simulink model "BaseEngineController_A02"  (Simulink 7.7)
settings: Solver=FixedStepDiscrete  StartTime=0.0  StopTime=10.0  RelTol=1e-3
PreLoadFcn:
  | try, eval([bdroot '_setup']); catch, eval('model_setup', ''); end
InitFcn:
  | try, eval([bdroot '_setup']); catch, eval('model_setup', ''); end
undefined symbols (not defined in the file or in its folder): ECU555, High, motohawk_build_version, motohawk_get_faults, motohawk_trunc_name, motohawk_vardec_config_group, motohawk_vardec_path, True
libraries: MotoHawk_lib x1145, AutoDoc_lib x786, motohawk_control_primitives_lib x114, motohawk_engine_lib x19, motohawk_annotations_lib x12, simulink x11, simulink_extras x1
(part 13 of 23)

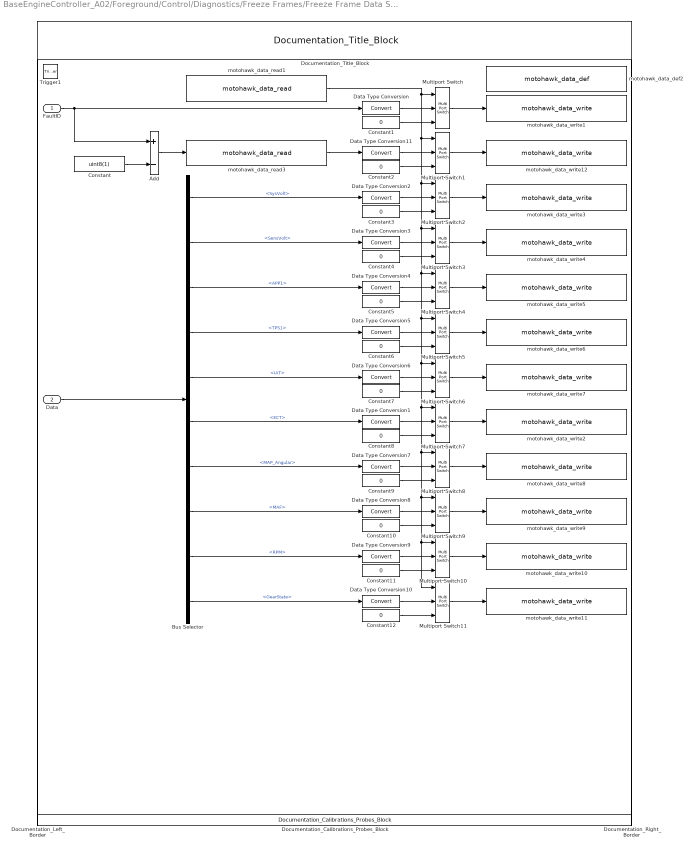
== system BaseEngineController_A02/Foreground/Control/Diagnostics/Freeze Frames/Freeze Frame Data Storage1 ==
  FaultID : Inport
  Data : Inport  Port=2
  Trigger1 : TriggerPort
  Add : Sum  Inputs=+-  OutDataTypeStr=Inherit: Same as first input
  Bus Selector : BusSelector  OutputSignals=SynthSensors.Raw.LinearSensors.SysVolt,SynthSensors.Raw.LinearSensors.SensVolt,SynthSensors.Raw.PotSensors.APP1,SynthSensors.Raw.PotSensors.TPS1,SynthSensors.Raw.NonLinearSensors.IAT,SynthSensors.Raw.NonLinearSensors.ECT,SynthSensors.Raw.LinearSensors.MAP_Angular,SynthSensors.Raw.Digital.MAF,SynthSensors.Raw.RPM,SynthSensors.Raw.Digital.GearState
  Constant : Constant  Value=uint8(1)
  Constant{1..12} (12 alike) : Constant  Value=0  OutDataTypeStr=Inherit: Inherit via back propagation
  Data Type Conversion{,1..11} (12 alike) : DataTypeConversion
  Documentation_Calibrations_Probes_Block : AutoDoc_lib/Documentation_Calibrations_Probes_Block
  Documentation_Left_Border : AutoDoc_lib/Documentation_Left_Border  layout=Portrait
  Documentation_Right_Border : AutoDoc_lib/Documentation_Right_Border  layout=Portrait
  Documentation_Title_Block : AutoDoc_lib/Documentation_Title_Block
  Multiport Switch{,1..11} (12 alike) : MultiPortSwitch  DataPortOrder=Zero-based contiguous  Inputs=2  zeroidx=on  InputSameDT=on
  motohawk_data_def2 : MotoHawk_lib/Data Storage Blocks/motohawk_data_def  nam=['FF' num2str(FFnum)]  container_nam='FreezeFramesContainer'  data=struct('Name', int32(0), 'Code', int32(0), 'Var1', int32(0), 'Var2', int32(0), 'Var3', int32(0), 'Var4', int32(0), 'Var5', int32(0), 'Var6', int32(0), 'Var7', int32(0), 'Var8', int32(0), 'Var9', int32(0), 'Var10', int32(0))  typ=struct  struct_nam='FreezeFrames'  storage=NonVolatile  outputRef=off  use_vardec=off  window=Display  read_level=1  write_level=(Read Only)  ovr_cals=on  view_as=Number  enum_struct={'Disabled', 'Enabled'}  vector_style=Wide Row  use_help_units=off  help=''  units=''  use_header_enum=off  row_header_enum={}  col_header_enum={}  use_min_max=off  min_val=-inf  max_val=inf  use_precision=off  precision=''  gain=1  offset=0  exp=1  use_mototune_group=off  mototune_group=''  eval_name=FF1  data_stores=motohawk_sfun_data_def_data_stores  struct_instances=motohawk_sfun_data_def_struct_instances  vardecs=motohawk_sfun_data_def_vardecs
  motohawk_data_read1 : MotoHawk_lib/Data Storage Blocks/motohawk_data_read  source=Lookup By Name  structure=Scalar  operation=Read scalar from location by index  sz_vec=3  sz_row=2  sz_col=3  typ=boolean  nam='ClearFreezeFrames'  checkNull=on  struct_inst_name='Struct1'  struct_nam='MyStruct'
  motohawk_data_read3 : MotoHawk_lib/Data Storage Blocks/motohawk_data_read  source=Lookup By Name  structure=Vector  operation=Read scalar from location by index  sz_vec=3  sz_row=2  sz_col=3  typ=uint16  nam='FltCodes'  checkNull=on  struct_inst_name='Struct1'  struct_nam='MyStruct'
  motohawk_data_write1 : MotoHawk_lib/Data Storage Blocks/motohawk_data_write  source=Lookup By Name in Structure  structure=Scalar  operation=Write entire data structure at once  sz_vec=len  sz_row=10  sz_col=1  typ=int32  nam='Name'  checkNull=on  write_read_only=off  struct_inst_name=['FF' num2str(FFnum)]  struct_name='FreezeFrames'
  motohawk_data_write10 : MotoHawk_lib/Data Storage Blocks/motohawk_data_write  source=Lookup By Name in Structure  structure=Scalar  operation=Write entire data structure at once  sz_vec=len  sz_row=10  sz_col=1  typ=int32  nam='Var9'  checkNull=on  write_read_only=off  struct_inst_name=['FF' num2str(FFnum)]  struct_name='FreezeFrames'
  motohawk_data_write11 : MotoHawk_lib/Data Storage Blocks/motohawk_data_write  source=Lookup By Name in Structure  structure=Scalar  operation=Write entire data structure at once  sz_vec=len  sz_row=10  sz_col=1  typ=int32  nam='Var10'  checkNull=on  write_read_only=off  struct_inst_name=['FF' num2str(FFnum)]  struct_name='FreezeFrames'
  motohawk_data_write12 : MotoHawk_lib/Data Storage Blocks/motohawk_data_write  source=Lookup By Name in Structure  structure=Scalar  operation=Write entire data structure at once  sz_vec=len  sz_row=10  sz_col=1  typ=int32  nam='Code'  checkNull=on  write_read_only=off  struct_inst_name=['FF' num2str(FFnum)]  struct_name='FreezeFrames'
  motohawk_data_write2 : MotoHawk_lib/Data Storage Blocks/motohawk_data_write  source=Lookup By Name in Structure  structure=Scalar  operation=Write entire data structure at once  sz_vec=len  sz_row=10  sz_col=1  typ=int32  nam='Var6'  checkNull=on  write_read_only=off  struct_inst_name=['FF' num2str(FFnum)]  struct_name='FreezeFrames'
  motohawk_data_write3 : MotoHawk_lib/Data Storage Blocks/motohawk_data_write  source=Lookup By Name in Structure  structure=Scalar  operation=Write entire data structure at once  sz_vec=len  sz_row=10  sz_col=1  typ=int32  nam='Var1'  checkNull=on  write_read_only=off  struct_inst_name=['FF' num2str(FFnum)]  struct_name='FreezeFrames'
  motohawk_data_write4 : MotoHawk_lib/Data Storage Blocks/motohawk_data_write  source=Lookup By Name in Structure  structure=Scalar  operation=Write entire data structure at once  sz_vec=len  sz_row=10  sz_col=1  typ=int32  nam='Var2'  checkNull=on  write_read_only=off  struct_inst_name=['FF' num2str(FFnum)]  struct_name='FreezeFrames'
  motohawk_data_write5 : MotoHawk_lib/Data Storage Blocks/motohawk_data_write  source=Lookup By Name in Structure  structure=Scalar  operation=Write entire data structure at once  sz_vec=len  sz_row=10  sz_col=1  typ=int32  nam='Var3'  checkNull=on  write_read_only=off  struct_inst_name=['FF' num2str(FFnum)]  struct_name='FreezeFrames'
  motohawk_data_write6 : MotoHawk_lib/Data Storage Blocks/motohawk_data_write  source=Lookup By Name in Structure  structure=Scalar  operation=Write entire data structure at once  sz_vec=len  sz_row=10  sz_col=1  typ=int32  nam='Var4'  checkNull=on  write_read_only=off  struct_inst_name=['FF' num2str(FFnum)]  struct_name='FreezeFrames'
  motohawk_data_write7 : MotoHawk_lib/Data Storage Blocks/motohawk_data_write  source=Lookup By Name in Structure  structure=Scalar  operation=Write entire data structure at once  sz_vec=len  sz_row=10  sz_col=1  typ=int32  nam='Var5'  checkNull=on  write_read_only=off  struct_inst_name=['FF' num2str(FFnum)]  struct_name='FreezeFrames'
  motohawk_data_write8 : MotoHawk_lib/Data Storage Blocks/motohawk_data_write  source=Lookup By Name in Structure  structure=Scalar  operation=Write entire data structure at once  sz_vec=len  sz_row=10  sz_col=1  typ=int32  nam='Var7'  checkNull=on  write_read_only=off  struct_inst_name=['FF' num2str(FFnum)]  struct_name='FreezeFrames'
  motohawk_data_write9 : MotoHawk_lib/Data Storage Blocks/motohawk_data_write  source=Lookup By Name in Structure  structure=Scalar  operation=Write entire data structure at once  sz_vec=len  sz_row=10  sz_col=1  typ=int32  nam='Var8'  checkNull=on  write_read_only=off  struct_inst_name=['FF' num2str(FFnum)]  struct_name='FreezeFrames'
  wires:
  Data Type Conversion10/1 -> Multiport Switch11/2
  Data Type Conversion9/1 -> Multiport Switch10/2
  Data Type Conversion8/1 -> Multiport Switch9/2
  Data Type Conversion7/1 -> Multiport Switch8/2
  Data Type Conversion1/1 -> Multiport Switch7/2
  Data Type Conversion6/1 -> Multiport Switch6/2
  Data Type Conversion5/1 -> Multiport Switch5/2
  Data Type Conversion4/1 -> Multiport Switch4/2
  Data Type Conversion3/1 -> Multiport Switch3/2
  Data Type Conversion2/1 -> Multiport Switch2/2
  Multiport Switch11/1 -> motohawk_data_write11/1
  Multiport Switch10/1 -> motohawk_data_write10/1
  Multiport Switch9/1 -> motohawk_data_write9/1
  Multiport Switch8/1 -> motohawk_data_write8/1
  Multiport Switch7/1 -> motohawk_data_write2/1
  Multiport Switch6/1 -> motohawk_data_write7/1
  Multiport Switch5/1 -> motohawk_data_write6/1
  Multiport Switch4/1 -> motohawk_data_write5/1
  Multiport Switch3/1 -> motohawk_data_write4/1
  Multiport Switch2/1 -> motohawk_data_write3/1
  Constant12/1 -> Multiport Switch11/3
  Constant11/1 -> Multiport Switch10/3
  Constant10/1 -> Multiport Switch9/3
  Constant9/1 -> Multiport Switch8/3
  Constant8/1 -> Multiport Switch7/3
  Constant7/1 -> Multiport Switch6/3
  Constant6/1 -> Multiport Switch5/3
  Constant5/1 -> Multiport Switch4/3
  Constant4/1 -> Multiport Switch3/3
  Constant3/1 -> Multiport Switch2/3
  Data Type Conversion11/1 -> Multiport Switch1/2
  Constant2/1 -> Multiport Switch1/3
  Multiport Switch1/1 -> motohawk_data_write12/1
  Multiport Switch/1 -> motohawk_data_write1/1
  motohawk_data_read1/1 -> Multiport Switch10/1, Multiport Switch11/1, Multiport Switch9/1, Multiport Switch8/1, Multiport Switch7/1, Multiport Switch6/1, Multiport Switch5/1, Multiport Switch4/1, Multiport Switch3/1, Multiport Switch2/1, Multiport Switch1/1, Multiport Switch/1
  Constant1/1 -> Multiport Switch/3
  Data Type Conversion/1 -> Multiport Switch/2
  Add/1 -> motohawk_data_read3/1
  Constant/1 -> Add/2
  motohawk_data_read3/1 -> Data Type Conversion11/1
  Bus Selector/10 -> Data Type Conversion10/1  "<GearState>"
  Bus Selector/9 -> Data Type Conversion9/1  "<RPM>"
  Bus Selector/8 -> Data Type Conversion8/1  "<MAF>"
  Bus Selector/7 -> Data Type Conversion7/1  "<MAP_Angular>"
  Bus Selector/6 -> Data Type Conversion1/1  "<ECT>"
  Data/1 -> Bus Selector/1
  Bus Selector/5 -> Data Type Conversion6/1  "<IAT>"
  Bus Selector/4 -> Data Type Conversion5/1  "<TPS1>"
  Bus Selector/3 -> Data Type Conversion4/1  "<APP1>"
  Bus Selector/2 -> Data Type Conversion3/1  "<SensVolt>"
  Bus Selector/1 -> Data Type Conversion2/1  "<SysVolt>"
  FaultID/1 -> Add/1, Data Type Conversion/1

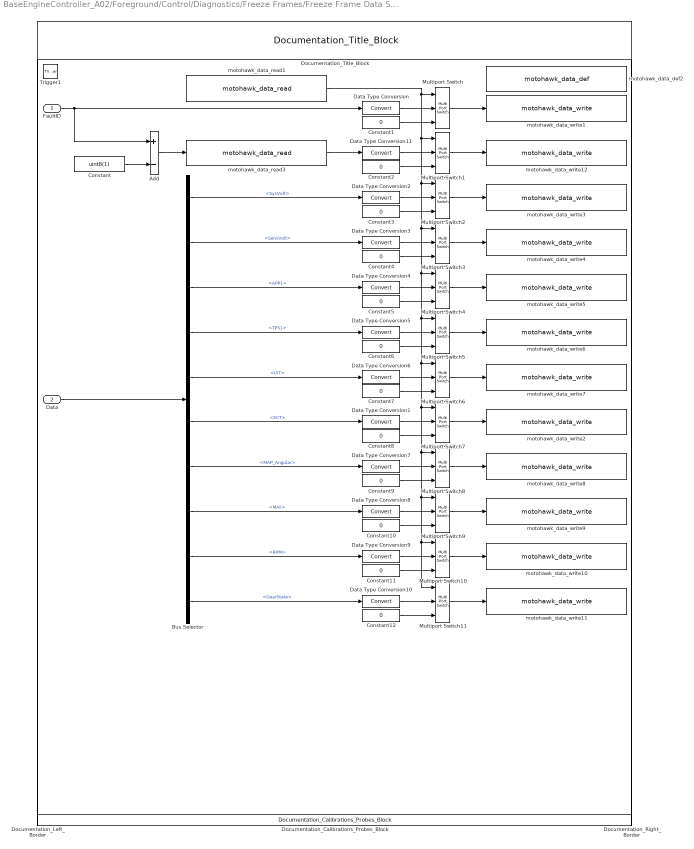
== system BaseEngineController_A02/Foreground/Control/Diagnostics/Freeze Frames/Freeze Frame Data Storage2 ==
  FaultID : Inport
  Data : Inport  Port=2
  Trigger1 : TriggerPort
  Add : Sum  Inputs=+-  OutDataTypeStr=Inherit: Same as first input
  Bus Selector : BusSelector  OutputSignals=SynthSensors.Raw.LinearSensors.SysVolt,SynthSensors.Raw.LinearSensors.SensVolt,SynthSensors.Raw.PotSensors.APP1,SynthSensors.Raw.PotSensors.TPS1,SynthSensors.Raw.NonLinearSensors.IAT,SynthSensors.Raw.NonLinearSensors.ECT,SynthSensors.Raw.LinearSensors.MAP_Angular,SynthSensors.Raw.Digital.MAF,SynthSensors.Raw.RPM,SynthSensors.Raw.Digital.GearState
  Constant : Constant  Value=uint8(1)
  Constant{1..12} (12 alike) : Constant  Value=0  OutDataTypeStr=Inherit: Inherit via back propagation
  Data Type Conversion{,1..11} (12 alike) : DataTypeConversion
  Documentation_Calibrations_Probes_Block : AutoDoc_lib/Documentation_Calibrations_Probes_Block
  Documentation_Left_Border : AutoDoc_lib/Documentation_Left_Border  layout=Portrait
  Documentation_Right_Border : AutoDoc_lib/Documentation_Right_Border  layout=Portrait
  Documentation_Title_Block : AutoDoc_lib/Documentation_Title_Block
  Multiport Switch{,1..11} (12 alike) : MultiPortSwitch  DataPortOrder=Zero-based contiguous  Inputs=2  zeroidx=on  InputSameDT=on
  motohawk_data_def2 : MotoHawk_lib/Data Storage Blocks/motohawk_data_def  nam=['FF' num2str(FFnum)]  container_nam='FreezeFramesContainer'  data=struct('Name', int32(0), 'Code', int32(0), 'Var1', int32(0), 'Var2', int32(0), 'Var3', int32(0), 'Var4', int32(0), 'Var5', int32(0), 'Var6', int32(0), 'Var7', int32(0), 'Var8', int32(0), 'Var9', int32(0), 'Var10', int32(0))  typ=struct  struct_nam='FreezeFrames'  storage=NonVolatile  outputRef=off  use_vardec=off  window=Display  read_level=1  write_level=(Read Only)  ovr_cals=on  view_as=Number  enum_struct={'Disabled', 'Enabled'}  vector_style=Wide Row  use_help_units=off  help=''  units=''  use_header_enum=off  row_header_enum={}  col_header_enum={}  use_min_max=off  min_val=-inf  max_val=inf  use_precision=off  precision=''  gain=1  offset=0  exp=1  use_mototune_group=off  mototune_group=''  eval_name=FF1  data_stores=motohawk_sfun_data_def_data_stores  struct_instances=motohawk_sfun_data_def_struct_instances  vardecs=motohawk_sfun_data_def_vardecs
  motohawk_data_read1 : MotoHawk_lib/Data Storage Blocks/motohawk_data_read  source=Lookup By Name  structure=Scalar  operation=Read scalar from location by index  sz_vec=3  sz_row=2  sz_col=3  typ=boolean  nam='ClearFreezeFrames'  checkNull=on  struct_inst_name='Struct1'  struct_nam='MyStruct'
  motohawk_data_read3 : MotoHawk_lib/Data Storage Blocks/motohawk_data_read  source=Lookup By Name  structure=Vector  operation=Read scalar from location by index  sz_vec=3  sz_row=2  sz_col=3  typ=uint16  nam='FltCodes'  checkNull=on  struct_inst_name='Struct1'  struct_nam='MyStruct'
  motohawk_data_write1 : MotoHawk_lib/Data Storage Blocks/motohawk_data_write  source=Lookup By Name in Structure  structure=Scalar  operation=Write entire data structure at once  sz_vec=len  sz_row=10  sz_col=1  typ=int32  nam='Name'  checkNull=on  write_read_only=off  struct_inst_name=['FF' num2str(FFnum)]  struct_name='FreezeFrames'
  motohawk_data_write10 : MotoHawk_lib/Data Storage Blocks/motohawk_data_write  source=Lookup By Name in Structure  structure=Scalar  operation=Write entire data structure at once  sz_vec=len  sz_row=10  sz_col=1  typ=int32  nam='Var9'  checkNull=on  write_read_only=off  struct_inst_name=['FF' num2str(FFnum)]  struct_name='FreezeFrames'
  motohawk_data_write11 : MotoHawk_lib/Data Storage Blocks/motohawk_data_write  source=Lookup By Name in Structure  structure=Scalar  operation=Write entire data structure at once  sz_vec=len  sz_row=10  sz_col=1  typ=int32  nam='Var10'  checkNull=on  write_read_only=off  struct_inst_name=['FF' num2str(FFnum)]  struct_name='FreezeFrames'
  motohawk_data_write12 : MotoHawk_lib/Data Storage Blocks/motohawk_data_write  source=Lookup By Name in Structure  structure=Scalar  operation=Write entire data structure at once  sz_vec=len  sz_row=10  sz_col=1  typ=int32  nam='Code'  checkNull=on  write_read_only=off  struct_inst_name=['FF' num2str(FFnum)]  struct_name='FreezeFrames'
  motohawk_data_write2 : MotoHawk_lib/Data Storage Blocks/motohawk_data_write  source=Lookup By Name in Structure  structure=Scalar  operation=Write entire data structure at once  sz_vec=len  sz_row=10  sz_col=1  typ=int32  nam='Var6'  checkNull=on  write_read_only=off  struct_inst_name=['FF' num2str(FFnum)]  struct_name='FreezeFrames'
  motohawk_data_write3 : MotoHawk_lib/Data Storage Blocks/motohawk_data_write  source=Lookup By Name in Structure  structure=Scalar  operation=Write entire data structure at once  sz_vec=len  sz_row=10  sz_col=1  typ=int32  nam='Var1'  checkNull=on  write_read_only=off  struct_inst_name=['FF' num2str(FFnum)]  struct_name='FreezeFrames'
  motohawk_data_write4 : MotoHawk_lib/Data Storage Blocks/motohawk_data_write  source=Lookup By Name in Structure  structure=Scalar  operation=Write entire data structure at once  sz_vec=len  sz_row=10  sz_col=1  typ=int32  nam='Var2'  checkNull=on  write_read_only=off  struct_inst_name=['FF' num2str(FFnum)]  struct_name='FreezeFrames'
  motohawk_data_write5 : MotoHawk_lib/Data Storage Blocks/motohawk_data_write  source=Lookup By Name in Structure  structure=Scalar  operation=Write entire data structure at once  sz_vec=len  sz_row=10  sz_col=1  typ=int32  nam='Var3'  checkNull=on  write_read_only=off  struct_inst_name=['FF' num2str(FFnum)]  struct_name='FreezeFrames'
  motohawk_data_write6 : MotoHawk_lib/Data Storage Blocks/motohawk_data_write  source=Lookup By Name in Structure  structure=Scalar  operation=Write entire data structure at once  sz_vec=len  sz_row=10  sz_col=1  typ=int32  nam='Var4'  checkNull=on  write_read_only=off  struct_inst_name=['FF' num2str(FFnum)]  struct_name='FreezeFrames'
  motohawk_data_write7 : MotoHawk_lib/Data Storage Blocks/motohawk_data_write  source=Lookup By Name in Structure  structure=Scalar  operation=Write entire data structure at once  sz_vec=len  sz_row=10  sz_col=1  typ=int32  nam='Var5'  checkNull=on  write_read_only=off  struct_inst_name=['FF' num2str(FFnum)]  struct_name='FreezeFrames'
  motohawk_data_write8 : MotoHawk_lib/Data Storage Blocks/motohawk_data_write  source=Lookup By Name in Structure  structure=Scalar  operation=Write entire data structure at once  sz_vec=len  sz_row=10  sz_col=1  typ=int32  nam='Var7'  checkNull=on  write_read_only=off  struct_inst_name=['FF' num2str(FFnum)]  struct_name='FreezeFrames'
  motohawk_data_write9 : MotoHawk_lib/Data Storage Blocks/motohawk_data_write  source=Lookup By Name in Structure  structure=Scalar  operation=Write entire data structure at once  sz_vec=len  sz_row=10  sz_col=1  typ=int32  nam='Var8'  checkNull=on  write_read_only=off  struct_inst_name=['FF' num2str(FFnum)]  struct_name='FreezeFrames'
  wires:
  FaultID/1 -> Data Type Conversion/1, Add/1
  Bus Selector/1 -> Data Type Conversion2/1  "<SysVolt>"
  Bus Selector/2 -> Data Type Conversion3/1  "<SensVolt>"
  Bus Selector/3 -> Data Type Conversion4/1  "<APP1>"
  Bus Selector/4 -> Data Type Conversion5/1  "<TPS1>"
  Bus Selector/5 -> Data Type Conversion6/1  "<IAT>"
  Data/1 -> Bus Selector/1
  Bus Selector/6 -> Data Type Conversion1/1  "<ECT>"
  Bus Selector/7 -> Data Type Conversion7/1  "<MAP_Angular>"
  Bus Selector/8 -> Data Type Conversion8/1  "<MAF>"
  Bus Selector/9 -> Data Type Conversion9/1  "<RPM>"
  Bus Selector/10 -> Data Type Conversion10/1  "<GearState>"
  motohawk_data_read3/1 -> Data Type Conversion11/1
  Constant/1 -> Add/2
  Add/1 -> motohawk_data_read3/1
  Data Type Conversion/1 -> Multiport Switch/2
  Constant1/1 -> Multiport Switch/3
  motohawk_data_read1/1 -> Multiport Switch/1, Multiport Switch1/1, Multiport Switch2/1, Multiport Switch3/1, Multiport Switch4/1, Multiport Switch5/1, Multiport Switch6/1, Multiport Switch7/1, Multiport Switch8/1, Multiport Switch9/1, Multiport Switch11/1, Multiport Switch10/1
  Multiport Switch/1 -> motohawk_data_write1/1
  Multiport Switch1/1 -> motohawk_data_write12/1
  Constant2/1 -> Multiport Switch1/3
  Data Type Conversion11/1 -> Multiport Switch1/2
  Constant3/1 -> Multiport Switch2/3
  Constant4/1 -> Multiport Switch3/3
  Constant5/1 -> Multiport Switch4/3
  Constant6/1 -> Multiport Switch5/3
  Constant7/1 -> Multiport Switch6/3
  Constant8/1 -> Multiport Switch7/3
  Constant9/1 -> Multiport Switch8/3
  Constant10/1 -> Multiport Switch9/3
  Constant11/1 -> Multiport Switch10/3
  Constant12/1 -> Multiport Switch11/3
  Multiport Switch2/1 -> motohawk_data_write3/1
  Multiport Switch3/1 -> motohawk_data_write4/1
  Multiport Switch4/1 -> motohawk_data_write5/1
  Multiport Switch5/1 -> motohawk_data_write6/1
  Multiport Switch6/1 -> motohawk_data_write7/1
  Multiport Switch7/1 -> motohawk_data_write2/1
  Multiport Switch8/1 -> motohawk_data_write8/1
  Multiport Switch9/1 -> motohawk_data_write9/1
  Multiport Switch10/1 -> motohawk_data_write10/1
  Multiport Switch11/1 -> motohawk_data_write11/1
  Data Type Conversion2/1 -> Multiport Switch2/2
  Data Type Conversion3/1 -> Multiport Switch3/2
  Data Type Conversion4/1 -> Multiport Switch4/2
  Data Type Conversion5/1 -> Multiport Switch5/2
  Data Type Conversion6/1 -> Multiport Switch6/2
  Data Type Conversion1/1 -> Multiport Switch7/2
  Data Type Conversion7/1 -> Multiport Switch8/2
  Data Type Conversion8/1 -> Multiport Switch9/2
  Data Type Conversion9/1 -> Multiport Switch10/2
  Data Type Conversion10/1 -> Multiport Switch11/2

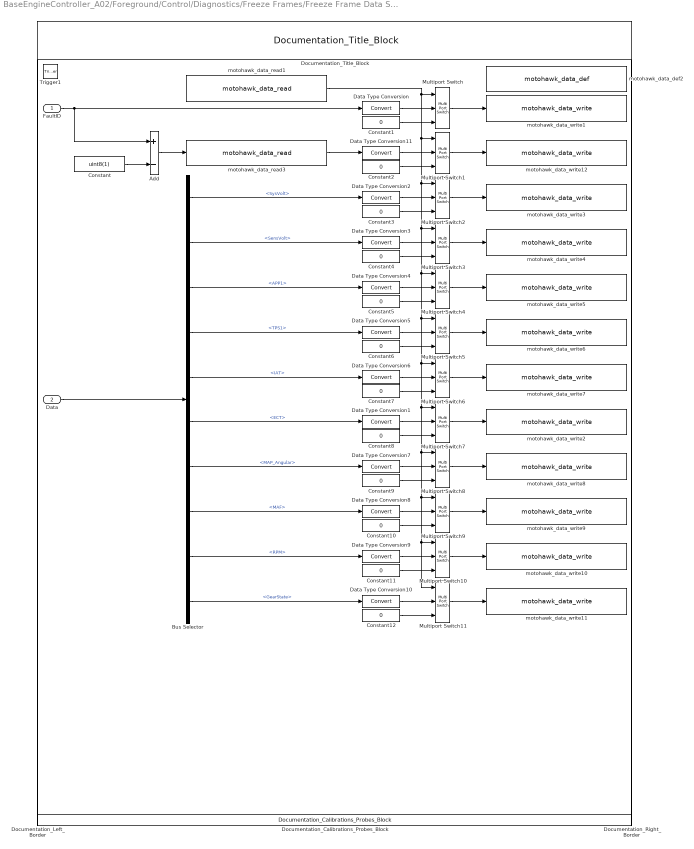
== system BaseEngineController_A02/Foreground/Control/Diagnostics/Freeze Frames/Freeze Frame Data Storage3 ==
  FaultID : Inport
  Data : Inport  Port=2
  Trigger1 : TriggerPort
  Add : Sum  Inputs=+-  OutDataTypeStr=Inherit: Same as first input
  Bus Selector : BusSelector  OutputSignals=SynthSensors.Raw.LinearSensors.SysVolt,SynthSensors.Raw.LinearSensors.SensVolt,SynthSensors.Raw.PotSensors.APP1,SynthSensors.Raw.PotSensors.TPS1,SynthSensors.Raw.NonLinearSensors.IAT,SynthSensors.Raw.NonLinearSensors.ECT,SynthSensors.Raw.LinearSensors.MAP_Angular,SynthSensors.Raw.Digital.MAF,SynthSensors.Raw.RPM,SynthSensors.Raw.Digital.GearState
  Constant : Constant  Value=uint8(1)
  Constant{1..12} (12 alike) : Constant  Value=0  OutDataTypeStr=Inherit: Inherit via back propagation
  Data Type Conversion{,1..11} (12 alike) : DataTypeConversion
  Documentation_Calibrations_Probes_Block : AutoDoc_lib/Documentation_Calibrations_Probes_Block
  Documentation_Left_Border : AutoDoc_lib/Documentation_Left_Border  layout=Portrait
  Documentation_Right_Border : AutoDoc_lib/Documentation_Right_Border  layout=Portrait
  Documentation_Title_Block : AutoDoc_lib/Documentation_Title_Block
  Multiport Switch{,1..11} (12 alike) : MultiPortSwitch  DataPortOrder=Zero-based contiguous  Inputs=2  zeroidx=on  InputSameDT=on
  motohawk_data_def2 : MotoHawk_lib/Data Storage Blocks/motohawk_data_def  nam=['FF' num2str(FFnum)]  container_nam='FreezeFramesContainer'  data=struct('Name', int32(0), 'Code', int32(0), 'Var1', int32(0), 'Var2', int32(0), 'Var3', int32(0), 'Var4', int32(0), 'Var5', int32(0), 'Var6', int32(0), 'Var7', int32(0), 'Var8', int32(0), 'Var9', int32(0), 'Var10', int32(0))  typ=struct  struct_nam='FreezeFrames'  storage=NonVolatile  outputRef=off  use_vardec=off  window=Display  read_level=1  write_level=(Read Only)  ovr_cals=on  view_as=Number  enum_struct={'Disabled', 'Enabled'}  vector_style=Wide Row  use_help_units=off  help=''  units=''  use_header_enum=off  row_header_enum={}  col_header_enum={}  use_min_max=off  min_val=-inf  max_val=inf  use_precision=off  precision=''  gain=1  offset=0  exp=1  use_mototune_group=off  mototune_group=''  eval_name=FF1  data_stores=motohawk_sfun_data_def_data_stores  struct_instances=motohawk_sfun_data_def_struct_instances  vardecs=motohawk_sfun_data_def_vardecs
  motohawk_data_read1 : MotoHawk_lib/Data Storage Blocks/motohawk_data_read  source=Lookup By Name  structure=Scalar  operation=Read scalar from location by index  sz_vec=3  sz_row=2  sz_col=3  typ=boolean  nam='ClearFreezeFrames'  checkNull=on  struct_inst_name='Struct1'  struct_nam='MyStruct'
  motohawk_data_read3 : MotoHawk_lib/Data Storage Blocks/motohawk_data_read  source=Lookup By Name  structure=Vector  operation=Read scalar from location by index  sz_vec=3  sz_row=2  sz_col=3  typ=uint16  nam='FltCodes'  checkNull=on  struct_inst_name='Struct1'  struct_nam='MyStruct'
  motohawk_data_write1 : MotoHawk_lib/Data Storage Blocks/motohawk_data_write  source=Lookup By Name in Structure  structure=Scalar  operation=Write entire data structure at once  sz_vec=len  sz_row=10  sz_col=1  typ=int32  nam='Name'  checkNull=on  write_read_only=off  struct_inst_name=['FF' num2str(FFnum)]  struct_name='FreezeFrames'
  motohawk_data_write10 : MotoHawk_lib/Data Storage Blocks/motohawk_data_write  source=Lookup By Name in Structure  structure=Scalar  operation=Write entire data structure at once  sz_vec=len  sz_row=10  sz_col=1  typ=int32  nam='Var9'  checkNull=on  write_read_only=off  struct_inst_name=['FF' num2str(FFnum)]  struct_name='FreezeFrames'
  motohawk_data_write11 : MotoHawk_lib/Data Storage Blocks/motohawk_data_write  source=Lookup By Name in Structure  structure=Scalar  operation=Write entire data structure at once  sz_vec=len  sz_row=10  sz_col=1  typ=int32  nam='Var10'  checkNull=on  write_read_only=off  struct_inst_name=['FF' num2str(FFnum)]  struct_name='FreezeFrames'
  motohawk_data_write12 : MotoHawk_lib/Data Storage Blocks/motohawk_data_write  source=Lookup By Name in Structure  structure=Scalar  operation=Write entire data structure at once  sz_vec=len  sz_row=10  sz_col=1  typ=int32  nam='Code'  checkNull=on  write_read_only=off  struct_inst_name=['FF' num2str(FFnum)]  struct_name='FreezeFrames'
  motohawk_data_write2 : MotoHawk_lib/Data Storage Blocks/motohawk_data_write  source=Lookup By Name in Structure  structure=Scalar  operation=Write entire data structure at once  sz_vec=len  sz_row=10  sz_col=1  typ=int32  nam='Var6'  checkNull=on  write_read_only=off  struct_inst_name=['FF' num2str(FFnum)]  struct_name='FreezeFrames'
  motohawk_data_write3 : MotoHawk_lib/Data Storage Blocks/motohawk_data_write  source=Lookup By Name in Structure  structure=Scalar  operation=Write entire data structure at once  sz_vec=len  sz_row=10  sz_col=1  typ=int32  nam='Var1'  checkNull=on  write_read_only=off  struct_inst_name=['FF' num2str(FFnum)]  struct_name='FreezeFrames'
  motohawk_data_write4 : MotoHawk_lib/Data Storage Blocks/motohawk_data_write  source=Lookup By Name in Structure  structure=Scalar  operation=Write entire data structure at once  sz_vec=len  sz_row=10  sz_col=1  typ=int32  nam='Var2'  checkNull=on  write_read_only=off  struct_inst_name=['FF' num2str(FFnum)]  struct_name='FreezeFrames'
  motohawk_data_write5 : MotoHawk_lib/Data Storage Blocks/motohawk_data_write  source=Lookup By Name in Structure  structure=Scalar  operation=Write entire data structure at once  sz_vec=len  sz_row=10  sz_col=1  typ=int32  nam='Var3'  checkNull=on  write_read_only=off  struct_inst_name=['FF' num2str(FFnum)]  struct_name='FreezeFrames'
  motohawk_data_write6 : MotoHawk_lib/Data Storage Blocks/motohawk_data_write  source=Lookup By Name in Structure  structure=Scalar  operation=Write entire data structure at once  sz_vec=len  sz_row=10  sz_col=1  typ=int32  nam='Var4'  checkNull=on  write_read_only=off  struct_inst_name=['FF' num2str(FFnum)]  struct_name='FreezeFrames'
  motohawk_data_write7 : MotoHawk_lib/Data Storage Blocks/motohawk_data_write  source=Lookup By Name in Structure  structure=Scalar  operation=Write entire data structure at once  sz_vec=len  sz_row=10  sz_col=1  typ=int32  nam='Var5'  checkNull=on  write_read_only=off  struct_inst_name=['FF' num2str(FFnum)]  struct_name='FreezeFrames'
  motohawk_data_write8 : MotoHawk_lib/Data Storage Blocks/motohawk_data_write  source=Lookup By Name in Structure  structure=Scalar  operation=Write entire data structure at once  sz_vec=len  sz_row=10  sz_col=1  typ=int32  nam='Var7'  checkNull=on  write_read_only=off  struct_inst_name=['FF' num2str(FFnum)]  struct_name='FreezeFrames'
  motohawk_data_write9 : MotoHawk_lib/Data Storage Blocks/motohawk_data_write  source=Lookup By Name in Structure  structure=Scalar  operation=Write entire data structure at once  sz_vec=len  sz_row=10  sz_col=1  typ=int32  nam='Var8'  checkNull=on  write_read_only=off  struct_inst_name=['FF' num2str(FFnum)]  struct_name='FreezeFrames'
  wires:
  Data Type Conversion10/1 -> Multiport Switch11/2
  Data Type Conversion9/1 -> Multiport Switch10/2
  Data Type Conversion8/1 -> Multiport Switch9/2
  Data Type Conversion7/1 -> Multiport Switch8/2
  Data Type Conversion1/1 -> Multiport Switch7/2
  Data Type Conversion6/1 -> Multiport Switch6/2
  Data Type Conversion5/1 -> Multiport Switch5/2
  Data Type Conversion4/1 -> Multiport Switch4/2
  Data Type Conversion3/1 -> Multiport Switch3/2
  Data Type Conversion2/1 -> Multiport Switch2/2
  Multiport Switch11/1 -> motohawk_data_write11/1
  Multiport Switch10/1 -> motohawk_data_write10/1
  Multiport Switch9/1 -> motohawk_data_write9/1
  Multiport Switch8/1 -> motohawk_data_write8/1
  Multiport Switch7/1 -> motohawk_data_write2/1
  Multiport Switch6/1 -> motohawk_data_write7/1
  Multiport Switch5/1 -> motohawk_data_write6/1
  Multiport Switch4/1 -> motohawk_data_write5/1
  Multiport Switch3/1 -> motohawk_data_write4/1
  Multiport Switch2/1 -> motohawk_data_write3/1
  Constant12/1 -> Multiport Switch11/3
  Constant11/1 -> Multiport Switch10/3
  Constant10/1 -> Multiport Switch9/3
  Constant9/1 -> Multiport Switch8/3
  Constant8/1 -> Multiport Switch7/3
  Constant7/1 -> Multiport Switch6/3
  Constant6/1 -> Multiport Switch5/3
  Constant5/1 -> Multiport Switch4/3
  Constant4/1 -> Multiport Switch3/3
  Constant3/1 -> Multiport Switch2/3
  Data Type Conversion11/1 -> Multiport Switch1/2
  Constant2/1 -> Multiport Switch1/3
  Multiport Switch1/1 -> motohawk_data_write12/1
  Multiport Switch/1 -> motohawk_data_write1/1
  motohawk_data_read1/1 -> Multiport Switch10/1, Multiport Switch11/1, Multiport Switch9/1, Multiport Switch8/1, Multiport Switch7/1, Multiport Switch6/1, Multiport Switch5/1, Multiport Switch4/1, Multiport Switch3/1, Multiport Switch2/1, Multiport Switch1/1, Multiport Switch/1
  Constant1/1 -> Multiport Switch/3
  Data Type Conversion/1 -> Multiport Switch/2
  Add/1 -> motohawk_data_read3/1
  Constant/1 -> Add/2
  motohawk_data_read3/1 -> Data Type Conversion11/1
  Bus Selector/10 -> Data Type Conversion10/1  "<GearState>"
  Bus Selector/9 -> Data Type Conversion9/1  "<RPM>"
  Bus Selector/8 -> Data Type Conversion8/1  "<MAF>"
  Bus Selector/7 -> Data Type Conversion7/1  "<MAP_Angular>"
  Bus Selector/6 -> Data Type Conversion1/1  "<ECT>"
  Data/1 -> Bus Selector/1
  Bus Selector/5 -> Data Type Conversion6/1  "<IAT>"
  Bus Selector/4 -> Data Type Conversion5/1  "<TPS1>"
  Bus Selector/3 -> Data Type Conversion4/1  "<APP1>"
  Bus Selector/2 -> Data Type Conversion3/1  "<SensVolt>"
  Bus Selector/1 -> Data Type Conversion2/1  "<SysVolt>"
  FaultID/1 -> Add/1, Data Type Conversion/1

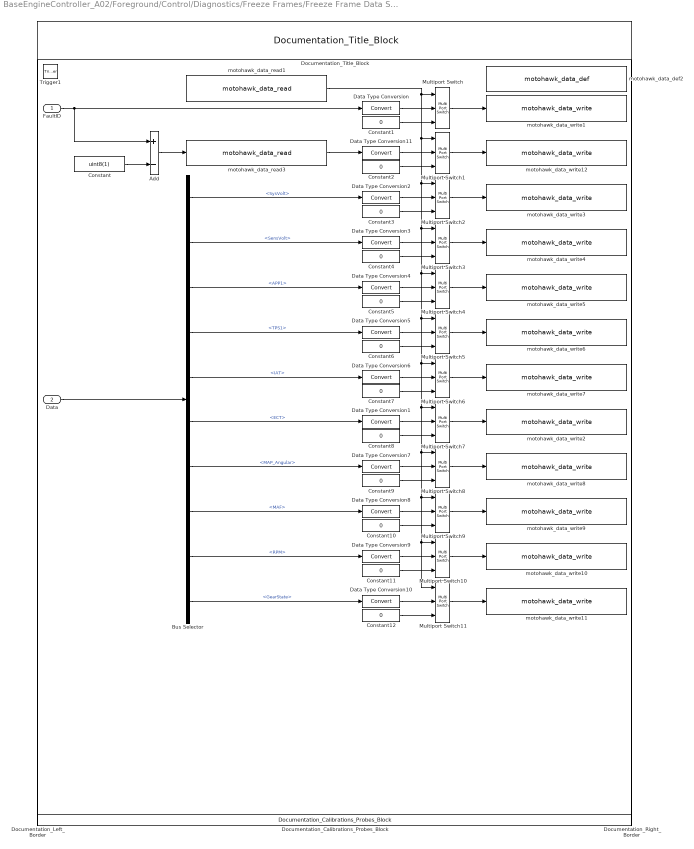
== system BaseEngineController_A02/Foreground/Control/Diagnostics/Freeze Frames/Freeze Frame Data Storage4 ==
  FaultID : Inport
  Data : Inport  Port=2
  Trigger1 : TriggerPort
  Add : Sum  Inputs=+-  OutDataTypeStr=Inherit: Same as first input
  Bus Selector : BusSelector  OutputSignals=SynthSensors.Raw.LinearSensors.SysVolt,SynthSensors.Raw.LinearSensors.SensVolt,SynthSensors.Raw.PotSensors.APP1,SynthSensors.Raw.PotSensors.TPS1,SynthSensors.Raw.NonLinearSensors.IAT,SynthSensors.Raw.NonLinearSensors.ECT,SynthSensors.Raw.LinearSensors.MAP_Angular,SynthSensors.Raw.Digital.MAF,SynthSensors.Raw.RPM,SynthSensors.Raw.Digital.GearState
  Constant : Constant  Value=uint8(1)
  Constant{1..12} (12 alike) : Constant  Value=0  OutDataTypeStr=Inherit: Inherit via back propagation
  Data Type Conversion{,1..11} (12 alike) : DataTypeConversion
  Documentation_Calibrations_Probes_Block : AutoDoc_lib/Documentation_Calibrations_Probes_Block
  Documentation_Left_Border : AutoDoc_lib/Documentation_Left_Border  layout=Portrait
  Documentation_Right_Border : AutoDoc_lib/Documentation_Right_Border  layout=Portrait
  Documentation_Title_Block : AutoDoc_lib/Documentation_Title_Block
  Multiport Switch{,1..11} (12 alike) : MultiPortSwitch  DataPortOrder=Zero-based contiguous  Inputs=2  zeroidx=on  InputSameDT=on
  motohawk_data_def2 : MotoHawk_lib/Data Storage Blocks/motohawk_data_def  nam=['FF' num2str(FFnum)]  container_nam='FreezeFramesContainer'  data=struct('Name', int32(0), 'Code', int32(0), 'Var1', int32(0), 'Var2', int32(0), 'Var3', int32(0), 'Var4', int32(0), 'Var5', int32(0), 'Var6', int32(0), 'Var7', int32(0), 'Var8', int32(0), 'Var9', int32(0), 'Var10', int32(0))  typ=struct  struct_nam='FreezeFrames'  storage=NonVolatile  outputRef=off  use_vardec=off  window=Display  read_level=1  write_level=(Read Only)  ovr_cals=on  view_as=Number  enum_struct={'Disabled', 'Enabled'}  vector_style=Wide Row  use_help_units=off  help=''  units=''  use_header_enum=off  row_header_enum={}  col_header_enum={}  use_min_max=off  min_val=-inf  max_val=inf  use_precision=off  precision=''  gain=1  offset=0  exp=1  use_mototune_group=off  mototune_group=''  eval_name=FF1  data_stores=motohawk_sfun_data_def_data_stores  struct_instances=motohawk_sfun_data_def_struct_instances  vardecs=motohawk_sfun_data_def_vardecs
  motohawk_data_read1 : MotoHawk_lib/Data Storage Blocks/motohawk_data_read  source=Lookup By Name  structure=Scalar  operation=Read scalar from location by index  sz_vec=3  sz_row=2  sz_col=3  typ=boolean  nam='ClearFreezeFrames'  checkNull=on  struct_inst_name='Struct1'  struct_nam='MyStruct'
  motohawk_data_read3 : MotoHawk_lib/Data Storage Blocks/motohawk_data_read  source=Lookup By Name  structure=Vector  operation=Read scalar from location by index  sz_vec=3  sz_row=2  sz_col=3  typ=uint16  nam='FltCodes'  checkNull=on  struct_inst_name='Struct1'  struct_nam='MyStruct'
  motohawk_data_write1 : MotoHawk_lib/Data Storage Blocks/motohawk_data_write  source=Lookup By Name in Structure  structure=Scalar  operation=Write entire data structure at once  sz_vec=len  sz_row=10  sz_col=1  typ=int32  nam='Name'  checkNull=on  write_read_only=off  struct_inst_name=['FF' num2str(FFnum)]  struct_name='FreezeFrames'
  motohawk_data_write10 : MotoHawk_lib/Data Storage Blocks/motohawk_data_write  source=Lookup By Name in Structure  structure=Scalar  operation=Write entire data structure at once  sz_vec=len  sz_row=10  sz_col=1  typ=int32  nam='Var9'  checkNull=on  write_read_only=off  struct_inst_name=['FF' num2str(FFnum)]  struct_name='FreezeFrames'
  motohawk_data_write11 : MotoHawk_lib/Data Storage Blocks/motohawk_data_write  source=Lookup By Name in Structure  structure=Scalar  operation=Write entire data structure at once  sz_vec=len  sz_row=10  sz_col=1  typ=int32  nam='Var10'  checkNull=on  write_read_only=off  struct_inst_name=['FF' num2str(FFnum)]  struct_name='FreezeFrames'
  motohawk_data_write12 : MotoHawk_lib/Data Storage Blocks/motohawk_data_write  source=Lookup By Name in Structure  structure=Scalar  operation=Write entire data structure at once  sz_vec=len  sz_row=10  sz_col=1  typ=int32  nam='Code'  checkNull=on  write_read_only=off  struct_inst_name=['FF' num2str(FFnum)]  struct_name='FreezeFrames'
  motohawk_data_write2 : MotoHawk_lib/Data Storage Blocks/motohawk_data_write  source=Lookup By Name in Structure  structure=Scalar  operation=Write entire data structure at once  sz_vec=len  sz_row=10  sz_col=1  typ=int32  nam='Var6'  checkNull=on  write_read_only=off  struct_inst_name=['FF' num2str(FFnum)]  struct_name='FreezeFrames'
  motohawk_data_write3 : MotoHawk_lib/Data Storage Blocks/motohawk_data_write  source=Lookup By Name in Structure  structure=Scalar  operation=Write entire data structure at once  sz_vec=len  sz_row=10  sz_col=1  typ=int32  nam='Var1'  checkNull=on  write_read_only=off  struct_inst_name=['FF' num2str(FFnum)]  struct_name='FreezeFrames'
  motohawk_data_write4 : MotoHawk_lib/Data Storage Blocks/motohawk_data_write  source=Lookup By Name in Structure  structure=Scalar  operation=Write entire data structure at once  sz_vec=len  sz_row=10  sz_col=1  typ=int32  nam='Var2'  checkNull=on  write_read_only=off  struct_inst_name=['FF' num2str(FFnum)]  struct_name='FreezeFrames'
  motohawk_data_write5 : MotoHawk_lib/Data Storage Blocks/motohawk_data_write  source=Lookup By Name in Structure  structure=Scalar  operation=Write entire data structure at once  sz_vec=len  sz_row=10  sz_col=1  typ=int32  nam='Var3'  checkNull=on  write_read_only=off  struct_inst_name=['FF' num2str(FFnum)]  struct_name='FreezeFrames'
  motohawk_data_write6 : MotoHawk_lib/Data Storage Blocks/motohawk_data_write  source=Lookup By Name in Structure  structure=Scalar  operation=Write entire data structure at once  sz_vec=len  sz_row=10  sz_col=1  typ=int32  nam='Var4'  checkNull=on  write_read_only=off  struct_inst_name=['FF' num2str(FFnum)]  struct_name='FreezeFrames'
  motohawk_data_write7 : MotoHawk_lib/Data Storage Blocks/motohawk_data_write  source=Lookup By Name in Structure  structure=Scalar  operation=Write entire data structure at once  sz_vec=len  sz_row=10  sz_col=1  typ=int32  nam='Var5'  checkNull=on  write_read_only=off  struct_inst_name=['FF' num2str(FFnum)]  struct_name='FreezeFrames'
  motohawk_data_write8 : MotoHawk_lib/Data Storage Blocks/motohawk_data_write  source=Lookup By Name in Structure  structure=Scalar  operation=Write entire data structure at once  sz_vec=len  sz_row=10  sz_col=1  typ=int32  nam='Var7'  checkNull=on  write_read_only=off  struct_inst_name=['FF' num2str(FFnum)]  struct_name='FreezeFrames'
  motohawk_data_write9 : MotoHawk_lib/Data Storage Blocks/motohawk_data_write  source=Lookup By Name in Structure  structure=Scalar  operation=Write entire data structure at once  sz_vec=len  sz_row=10  sz_col=1  typ=int32  nam='Var8'  checkNull=on  write_read_only=off  struct_inst_name=['FF' num2str(FFnum)]  struct_name='FreezeFrames'
  wires:
  FaultID/1 -> Data Type Conversion/1, Add/1
  Bus Selector/1 -> Data Type Conversion2/1  "<SysVolt>"
  Bus Selector/2 -> Data Type Conversion3/1  "<SensVolt>"
  Bus Selector/3 -> Data Type Conversion4/1  "<APP1>"
  Bus Selector/4 -> Data Type Conversion5/1  "<TPS1>"
  Bus Selector/5 -> Data Type Conversion6/1  "<IAT>"
  Data/1 -> Bus Selector/1
  Bus Selector/6 -> Data Type Conversion1/1  "<ECT>"
  Bus Selector/7 -> Data Type Conversion7/1  "<MAP_Angular>"
  Bus Selector/8 -> Data Type Conversion8/1  "<MAF>"
  Bus Selector/9 -> Data Type Conversion9/1  "<RPM>"
  Bus Selector/10 -> Data Type Conversion10/1  "<GearState>"
  motohawk_data_read3/1 -> Data Type Conversion11/1
  Constant/1 -> Add/2
  Add/1 -> motohawk_data_read3/1
  Data Type Conversion/1 -> Multiport Switch/2
  Constant1/1 -> Multiport Switch/3
  motohawk_data_read1/1 -> Multiport Switch/1, Multiport Switch1/1, Multiport Switch2/1, Multiport Switch3/1, Multiport Switch4/1, Multiport Switch5/1, Multiport Switch6/1, Multiport Switch7/1, Multiport Switch8/1, Multiport Switch9/1, Multiport Switch11/1, Multiport Switch10/1
  Multiport Switch/1 -> motohawk_data_write1/1
  Multiport Switch1/1 -> motohawk_data_write12/1
  Constant2/1 -> Multiport Switch1/3
  Data Type Conversion11/1 -> Multiport Switch1/2
  Constant3/1 -> Multiport Switch2/3
  Constant4/1 -> Multiport Switch3/3
  Constant5/1 -> Multiport Switch4/3
  Constant6/1 -> Multiport Switch5/3
  Constant7/1 -> Multiport Switch6/3
  Constant8/1 -> Multiport Switch7/3
  Constant9/1 -> Multiport Switch8/3
  Constant10/1 -> Multiport Switch9/3
  Constant11/1 -> Multiport Switch10/3
  Constant12/1 -> Multiport Switch11/3
  Multiport Switch2/1 -> motohawk_data_write3/1
  Multiport Switch3/1 -> motohawk_data_write4/1
  Multiport Switch4/1 -> motohawk_data_write5/1
  Multiport Switch5/1 -> motohawk_data_write6/1
  Multiport Switch6/1 -> motohawk_data_write7/1
  Multiport Switch7/1 -> motohawk_data_write2/1
  Multiport Switch8/1 -> motohawk_data_write8/1
  Multiport Switch9/1 -> motohawk_data_write9/1
  Multiport Switch10/1 -> motohawk_data_write10/1
  Multiport Switch11/1 -> motohawk_data_write11/1
  Data Type Conversion2/1 -> Multiport Switch2/2
  Data Type Conversion3/1 -> Multiport Switch3/2
  Data Type Conversion4/1 -> Multiport Switch4/2
  Data Type Conversion5/1 -> Multiport Switch5/2
  Data Type Conversion6/1 -> Multiport Switch6/2
  Data Type Conversion1/1 -> Multiport Switch7/2
  Data Type Conversion7/1 -> Multiport Switch8/2
  Data Type Conversion8/1 -> Multiport Switch9/2
  Data Type Conversion9/1 -> Multiport Switch10/2
  Data Type Conversion10/1 -> Multiport Switch11/2

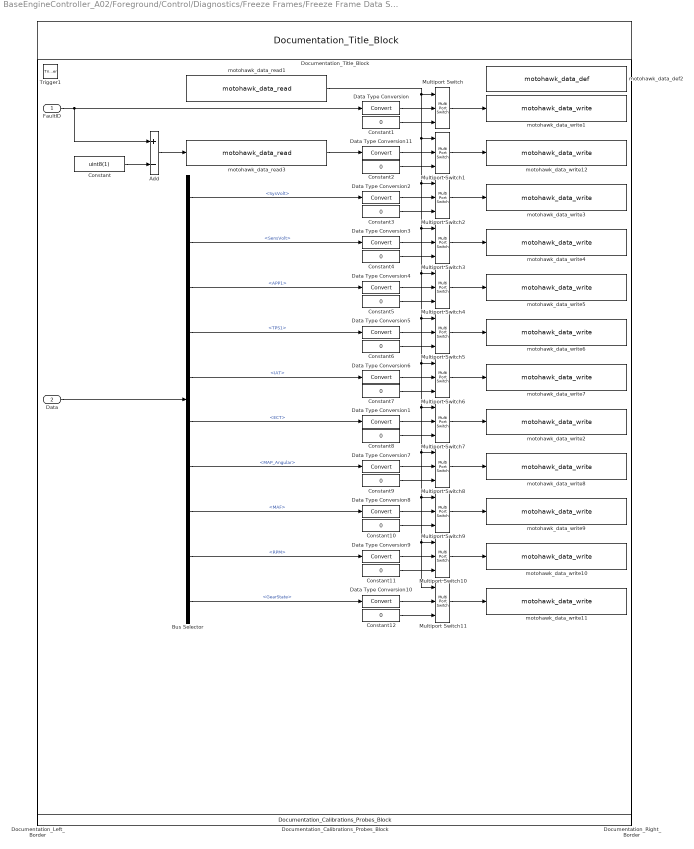
== system BaseEngineController_A02/Foreground/Control/Diagnostics/Freeze Frames/Freeze Frame Data Storage5 ==
  FaultID : Inport
  Data : Inport  Port=2
  Trigger1 : TriggerPort
  Add : Sum  Inputs=+-  OutDataTypeStr=Inherit: Same as first input
  Bus Selector : BusSelector  OutputSignals=SynthSensors.Raw.LinearSensors.SysVolt,SynthSensors.Raw.LinearSensors.SensVolt,SynthSensors.Raw.PotSensors.APP1,SynthSensors.Raw.PotSensors.TPS1,SynthSensors.Raw.NonLinearSensors.IAT,SynthSensors.Raw.NonLinearSensors.ECT,SynthSensors.Raw.LinearSensors.MAP_Angular,SynthSensors.Raw.Digital.MAF,SynthSensors.Raw.RPM,SynthSensors.Raw.Digital.GearState
  Constant : Constant  Value=uint8(1)
  Constant{1..12} (12 alike) : Constant  Value=0  OutDataTypeStr=Inherit: Inherit via back propagation
  Data Type Conversion{,1..11} (12 alike) : DataTypeConversion
  Documentation_Calibrations_Probes_Block : AutoDoc_lib/Documentation_Calibrations_Probes_Block
  Documentation_Left_Border : AutoDoc_lib/Documentation_Left_Border  layout=Portrait
  Documentation_Right_Border : AutoDoc_lib/Documentation_Right_Border  layout=Portrait
  Documentation_Title_Block : AutoDoc_lib/Documentation_Title_Block
  Multiport Switch{,1..11} (12 alike) : MultiPortSwitch  DataPortOrder=Zero-based contiguous  Inputs=2  zeroidx=on  InputSameDT=on
  motohawk_data_def2 : MotoHawk_lib/Data Storage Blocks/motohawk_data_def  nam=['FF' num2str(FFnum)]  container_nam='FreezeFramesContainer'  data=struct('Name', int32(0), 'Code', int32(0), 'Var1', int32(0), 'Var2', int32(0), 'Var3', int32(0), 'Var4', int32(0), 'Var5', int32(0), 'Var6', int32(0), 'Var7', int32(0), 'Var8', int32(0), 'Var9', int32(0), 'Var10', int32(0))  typ=struct  struct_nam='FreezeFrames'  storage=NonVolatile  outputRef=off  use_vardec=off  window=Display  read_level=1  write_level=(Read Only)  ovr_cals=on  view_as=Number  enum_struct={'Disabled', 'Enabled'}  vector_style=Wide Row  use_help_units=off  help=''  units=''  use_header_enum=off  row_header_enum={}  col_header_enum={}  use_min_max=off  min_val=-inf  max_val=inf  use_precision=off  precision=''  gain=1  offset=0  exp=1  use_mototune_group=off  mototune_group=''  eval_name=FF1  data_stores=motohawk_sfun_data_def_data_stores  struct_instances=motohawk_sfun_data_def_struct_instances  vardecs=motohawk_sfun_data_def_vardecs
  motohawk_data_read1 : MotoHawk_lib/Data Storage Blocks/motohawk_data_read  source=Lookup By Name  structure=Scalar  operation=Read scalar from location by index  sz_vec=3  sz_row=2  sz_col=3  typ=boolean  nam='ClearFreezeFrames'  checkNull=on  struct_inst_name='Struct1'  struct_nam='MyStruct'
  motohawk_data_read3 : MotoHawk_lib/Data Storage Blocks/motohawk_data_read  source=Lookup By Name  structure=Vector  operation=Read scalar from location by index  sz_vec=3  sz_row=2  sz_col=3  typ=uint16  nam='FltCodes'  checkNull=on  struct_inst_name='Struct1'  struct_nam='MyStruct'
  motohawk_data_write1 : MotoHawk_lib/Data Storage Blocks/motohawk_data_write  source=Lookup By Name in Structure  structure=Scalar  operation=Write entire data structure at once  sz_vec=len  sz_row=10  sz_col=1  typ=int32  nam='Name'  checkNull=on  write_read_only=off  struct_inst_name=['FF' num2str(FFnum)]  struct_name='FreezeFrames'
  motohawk_data_write10 : MotoHawk_lib/Data Storage Blocks/motohawk_data_write  source=Lookup By Name in Structure  structure=Scalar  operation=Write entire data structure at once  sz_vec=len  sz_row=10  sz_col=1  typ=int32  nam='Var9'  checkNull=on  write_read_only=off  struct_inst_name=['FF' num2str(FFnum)]  struct_name='FreezeFrames'
  motohawk_data_write11 : MotoHawk_lib/Data Storage Blocks/motohawk_data_write  source=Lookup By Name in Structure  structure=Scalar  operation=Write entire data structure at once  sz_vec=len  sz_row=10  sz_col=1  typ=int32  nam='Var10'  checkNull=on  write_read_only=off  struct_inst_name=['FF' num2str(FFnum)]  struct_name='FreezeFrames'
  motohawk_data_write12 : MotoHawk_lib/Data Storage Blocks/motohawk_data_write  source=Lookup By Name in Structure  structure=Scalar  operation=Write entire data structure at once  sz_vec=len  sz_row=10  sz_col=1  typ=int32  nam='Code'  checkNull=on  write_read_only=off  struct_inst_name=['FF' num2str(FFnum)]  struct_name='FreezeFrames'
  motohawk_data_write2 : MotoHawk_lib/Data Storage Blocks/motohawk_data_write  source=Lookup By Name in Structure  structure=Scalar  operation=Write entire data structure at once  sz_vec=len  sz_row=10  sz_col=1  typ=int32  nam='Var6'  checkNull=on  write_read_only=off  struct_inst_name=['FF' num2str(FFnum)]  struct_name='FreezeFrames'
  motohawk_data_write3 : MotoHawk_lib/Data Storage Blocks/motohawk_data_write  source=Lookup By Name in Structure  structure=Scalar  operation=Write entire data structure at once  sz_vec=len  sz_row=10  sz_col=1  typ=int32  nam='Var1'  checkNull=on  write_read_only=off  struct_inst_name=['FF' num2str(FFnum)]  struct_name='FreezeFrames'
  motohawk_data_write4 : MotoHawk_lib/Data Storage Blocks/motohawk_data_write  source=Lookup By Name in Structure  structure=Scalar  operation=Write entire data structure at once  sz_vec=len  sz_row=10  sz_col=1  typ=int32  nam='Var2'  checkNull=on  write_read_only=off  struct_inst_name=['FF' num2str(FFnum)]  struct_name='FreezeFrames'
  motohawk_data_write5 : MotoHawk_lib/Data Storage Blocks/motohawk_data_write  source=Lookup By Name in Structure  structure=Scalar  operation=Write entire data structure at once  sz_vec=len  sz_row=10  sz_col=1  typ=int32  nam='Var3'  checkNull=on  write_read_only=off  struct_inst_name=['FF' num2str(FFnum)]  struct_name='FreezeFrames'
  motohawk_data_write6 : MotoHawk_lib/Data Storage Blocks/motohawk_data_write  source=Lookup By Name in Structure  structure=Scalar  operation=Write entire data structure at once  sz_vec=len  sz_row=10  sz_col=1  typ=int32  nam='Var4'  checkNull=on  write_read_only=off  struct_inst_name=['FF' num2str(FFnum)]  struct_name='FreezeFrames'
  motohawk_data_write7 : MotoHawk_lib/Data Storage Blocks/motohawk_data_write  source=Lookup By Name in Structure  structure=Scalar  operation=Write entire data structure at once  sz_vec=len  sz_row=10  sz_col=1  typ=int32  nam='Var5'  checkNull=on  write_read_only=off  struct_inst_name=['FF' num2str(FFnum)]  struct_name='FreezeFrames'
  motohawk_data_write8 : MotoHawk_lib/Data Storage Blocks/motohawk_data_write  source=Lookup By Name in Structure  structure=Scalar  operation=Write entire data structure at once  sz_vec=len  sz_row=10  sz_col=1  typ=int32  nam='Var7'  checkNull=on  write_read_only=off  struct_inst_name=['FF' num2str(FFnum)]  struct_name='FreezeFrames'
  motohawk_data_write9 : MotoHawk_lib/Data Storage Blocks/motohawk_data_write  source=Lookup By Name in Structure  structure=Scalar  operation=Write entire data structure at once  sz_vec=len  sz_row=10  sz_col=1  typ=int32  nam='Var8'  checkNull=on  write_read_only=off  struct_inst_name=['FF' num2str(FFnum)]  struct_name='FreezeFrames'
  wires:
  Data Type Conversion10/1 -> Multiport Switch11/2
  Data Type Conversion9/1 -> Multiport Switch10/2
  Data Type Conversion8/1 -> Multiport Switch9/2
  Data Type Conversion7/1 -> Multiport Switch8/2
  Data Type Conversion1/1 -> Multiport Switch7/2
  Data Type Conversion6/1 -> Multiport Switch6/2
  Data Type Conversion5/1 -> Multiport Switch5/2
  Data Type Conversion4/1 -> Multiport Switch4/2
  Data Type Conversion3/1 -> Multiport Switch3/2
  Data Type Conversion2/1 -> Multiport Switch2/2
  Multiport Switch11/1 -> motohawk_data_write11/1
  Multiport Switch10/1 -> motohawk_data_write10/1
  Multiport Switch9/1 -> motohawk_data_write9/1
  Multiport Switch8/1 -> motohawk_data_write8/1
  Multiport Switch7/1 -> motohawk_data_write2/1
  Multiport Switch6/1 -> motohawk_data_write7/1
  Multiport Switch5/1 -> motohawk_data_write6/1
  Multiport Switch4/1 -> motohawk_data_write5/1
  Multiport Switch3/1 -> motohawk_data_write4/1
  Multiport Switch2/1 -> motohawk_data_write3/1
  Constant12/1 -> Multiport Switch11/3
  Constant11/1 -> Multiport Switch10/3
  Constant10/1 -> Multiport Switch9/3
  Constant9/1 -> Multiport Switch8/3
  Constant8/1 -> Multiport Switch7/3
  Constant7/1 -> Multiport Switch6/3
  Constant6/1 -> Multiport Switch5/3
  Constant5/1 -> Multiport Switch4/3
  Constant4/1 -> Multiport Switch3/3
  Constant3/1 -> Multiport Switch2/3
  Data Type Conversion11/1 -> Multiport Switch1/2
  Constant2/1 -> Multiport Switch1/3
  Multiport Switch1/1 -> motohawk_data_write12/1
  Multiport Switch/1 -> motohawk_data_write1/1
  motohawk_data_read1/1 -> Multiport Switch10/1, Multiport Switch11/1, Multiport Switch9/1, Multiport Switch8/1, Multiport Switch7/1, Multiport Switch6/1, Multiport Switch5/1, Multiport Switch4/1, Multiport Switch3/1, Multiport Switch2/1, Multiport Switch1/1, Multiport Switch/1
  Constant1/1 -> Multiport Switch/3
  Data Type Conversion/1 -> Multiport Switch/2
  Add/1 -> motohawk_data_read3/1
  Constant/1 -> Add/2
  motohawk_data_read3/1 -> Data Type Conversion11/1
  Bus Selector/10 -> Data Type Conversion10/1  "<GearState>"
  Bus Selector/9 -> Data Type Conversion9/1  "<RPM>"
  Bus Selector/8 -> Data Type Conversion8/1  "<MAF>"
  Bus Selector/7 -> Data Type Conversion7/1  "<MAP_Angular>"
  Bus Selector/6 -> Data Type Conversion1/1  "<ECT>"
  Data/1 -> Bus Selector/1
  Bus Selector/5 -> Data Type Conversion6/1  "<IAT>"
  Bus Selector/4 -> Data Type Conversion5/1  "<TPS1>"
  Bus Selector/3 -> Data Type Conversion4/1  "<APP1>"
  Bus Selector/2 -> Data Type Conversion3/1  "<SensVolt>"
  Bus Selector/1 -> Data Type Conversion2/1  "<SysVolt>"
  FaultID/1 -> Add/1, Data Type Conversion/1
